simulink model "CCMDcMotor"  (R2022b)
settings: Solver=FixedStepDiscrete  StartTime=0.0  StopTime=10.0  FixedStep=0.01  RelTol=1e-3
model workspace (MAT data in the model file):
  NvMBlockDcMtrCalData = Simulink.Signal
  NvMBlockDcMtrCalData.DataType = 'UInt8'
  NvMBlockDcMtrCalData.Dimensions = [82 1]
  NvMBlockDcMtrCalData.DimensionsMode = 'auto'
  NvMBlockDcMtrCalData.Complexity = 'real'
  NvMBlockDcMtrCalData.SampleTime = -1
  NvMBlockDcMtrCalData.SamplingMode = 'auto'
  NvMBlockDcMtrCalData.InitialValue = 'NvMBlockDcMtrCalDataInit'
  NvMBlockDcMtrCalDataInit = Simulink.Parameter
  NvMBlockDcMtrCalDataInit.Value = [0; 0; 0; 0; 0; 0; 0; 0; 0; 0; 0; 0; 0; 0; 0; 0; 0; 0; 0; 0; 0; 0; 0; 0; 0; 0; 0; 0; 0; 0; 0; 0; 0; 0; 0; 0; 0; 0; 0; 0; 0; 0; 0; 0; 0; 0; 0; 0; 0; 0; 0; 0; 0; 0; 0; 0; 0; 0; 0; 0; 0; 0; 0; 0; 0; 0; 0; 0; 0; 0; 0; 0; 0; 0; 0; 0; 0; 0; 0; 0; 0; 0]
  NvMBlockDcMtrCalDataInit.DataType = 'UInt8'
  NvMBlockDcMtrCalDataInit.Complexity = 'real'
  NvMBlockDcMtrCalDataInit.Dimensions = [82 1]
undefined symbols (not defined in the file or in its folder): DTCState, NvMBlockId, P_Std_ReturnType, Tle91ChlType, Tle91CmdType
(part 1 of 3)
settings Model: Creator=blue.ge  LastModifiedBy=blue.ge
settings DataIOCC: SaveFormat=StructureWithTime
settings OptimizationCC: DefaultParameterBehavior=Inlined  EnhancedBackFolding=on  PassReuseOutputArgsAs=Individual arguments  ZeroExternalMemoryAtStartup=off  ZeroInternalMemoryAtStartup=off  OptimizeBlockOrder=speed  DifferentSizesBufferReuse=on  OptimizationCustomize=off  EfficientTunableParamExpr=on
settings DebuggingCC: ParameterTunabilityLossMsg=error
settings ModelReferenceCC: ModelReferenceNumInstancesAllowed=Single
settings SFSimCC: MATLABDynamicMemAlloc=off
settings RTWCC: Description=AUTOSAR  SystemTargetFile=autosar.tlc  GenCodeOnly=on  TemplateMakefile=ert_default_tmf
settings CodeAppCC: ForceParamTrailComments=on  IgnoreCustomStorageClasses=off  OperatorAnnotations=on  SimulinkDataObjDesc=on  SFDataObjDesc=on  InsertBlockDesc=on
settings STFCustomTargetCC: TargetLangStandard=C99 (ISO)  MultiwordLength=256  ParMdlRefBuildCompliant=on  CompOptLevelCompliant=on  IncludeMdlTerminateFcn=off  CombineOutputUpdateFcns=on  SuppressErrorStatus=on  ERTCustomFileBanners=on  SupportNonFinite=off  SupportComplex=off  SupportContinuousTime=off  SupportNonInlinedSFcns=off  RemoveResetFunc=on  MATLABClassNameForMDSCustomization=Simulink.SoftwareTarget.ERTCustomization  AutosarCompliant=on  UseToolchainInfoCompliant=on  LookupTableObjectStructAxisOrder=2,1,3,4,...  LUTObjectStructOrderEvenSpacing=Size,Table,Breakpoints  SystemTargetFile=autosar.tlc
settings ERTTargetCC: TargetLangStandard=C99 (ISO)  ConcurrentExecutionCompliant=off  IncludeMdlTerminateFcn=off  SuppressErrorStatus=on  SupportNonFinite=off  SupportComplex=off  ModelStepFunctionPrototypeControlCompliant=off  CPPClassGenCompliant=off  AutosarCompliant=on  LookupTableObjectStructAxisOrder=2,1,3,4,...  LUTObjectStructOrderEvenSpacing=Size,Table,Breakpoints  InlinedParameterPlacement=NonHierarchical  ERTCustomFileTemplate=example_file_process.tlc

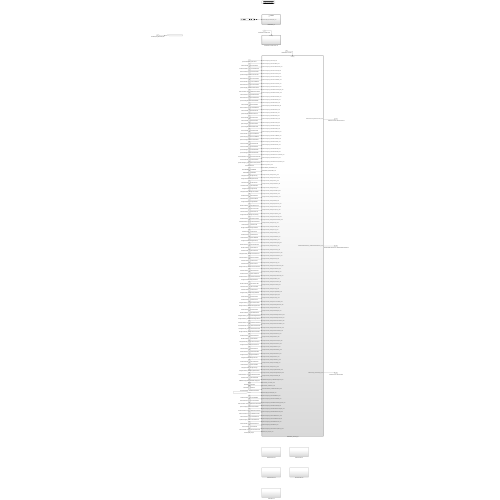
[thumbnail: Simulink's stored preview of the top-level diagram]
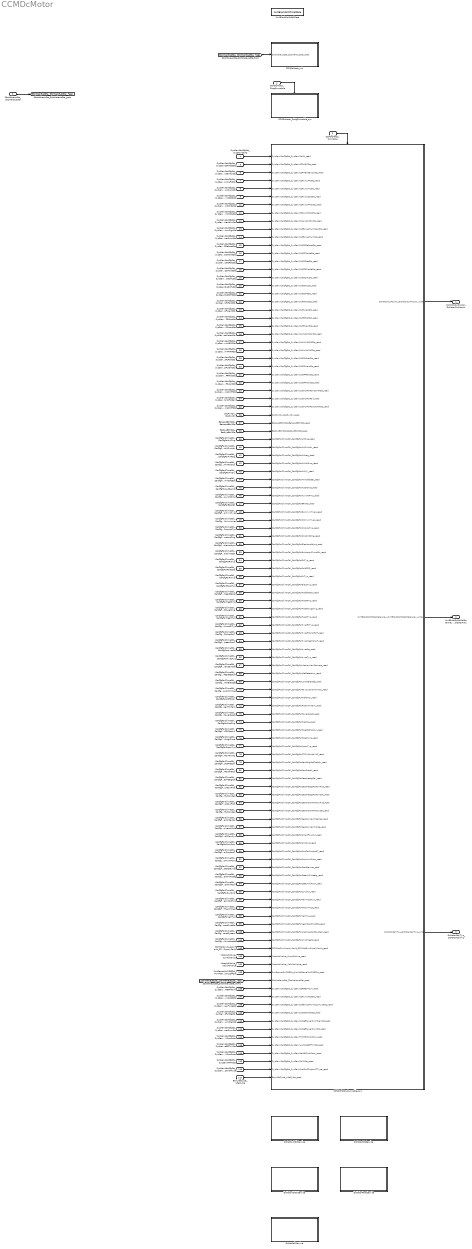
== system CCMDcMotor ==
  CCMDcMotor_DiagRunnable : Inport  SampleTime=0.05  OutputFunctionCall=on
  CCMDcMotor_Runnable : Inport  Port=2  SampleTime=0.01  OutputFunctionCall=on
  SbcWakeupSta_SbcWakeupSta : Inport  Port=3  OutDataTypeStr=Enum: SbcWakeupSta  PortDimensions=1
  SysServCarCfgSts_SysServCarID : Inport  Port=4  OutDataTypeStr=Enum: SysServCarID  PortDimensions=1
  SysServCarCfgSts_SysServCCPAQSSts : Inport  Port=5  OutDataTypeStr=UInt8  PortDimensions=1
  SysServCarCfgSts_SysServCCPHEXSensorSts : Inport  Port=6  OutDataTypeStr=UInt8  PortDimensions=1
  SysServCarCfgSts_SysServCCPLin2AFUSts : Inport  Port=7  OutDataTypeStr=Boolean  PortDimensions=1
  SysServCarCfgSts_SysServCCPLin2AIUSts : Inport  Port=8  OutDataTypeStr=UInt8  PortDimensions=1
  SysServCarCfgSts_SysServCCPLin2CDSSts : Inport  Port=9  OutDataTypeStr=Boolean  PortDimensions=1
  SysServCarCfgSts_SysServCCPLin2PMSISts : Inport  Port=10  OutDataTypeStr=Boolean  PortDimensions=1
  SysServCarCfgSts_SysServCCPLin3VGASts : Inport  Port=11  OutDataTypeStr=Boolean  PortDimensions=1
  SysServCarCfgSts_SysServDUAirVentSnrSts : Inport  Port=12  OutDataTypeStr=Boolean  PortDimensions=1
  SysServCarCfgSts_SysServDUBlowerPwmSignSts : Inport  Port=13  OutDataTypeStr=Boolean  PortDimensions=1
  SysServCarCfgSts_SysServDUBlowerPwmSts : Inport  Port=14  OutDataTypeStr=Boolean  PortDimensions=1
  SysServCarCfgSts_SysServDUDCDefrostSts : Inport  Port=15  OutDataTypeStr=UInt8  PortDimensions=1
  SysServCarCfgSts_SysServDUDCModeSts : Inport  Port=16  OutDataTypeStr=UInt8  PortDimensions=1
  SysServCarCfgSts_SysServDUDCRecSts : Inport  Port=17  OutDataTypeStr=UInt8  PortDimensions=1
  SysServCarCfgSts_SysServDUDCSModeSts : Inport  Port=18  OutDataTypeStr=UInt8  PortDimensions=1
  SysServCarCfgSts_SysServDUDCSTLSts : Inport  Port=19  OutDataTypeStr=UInt8  PortDimensions=1
  SysServCarCfgSts_SysServDUDCTLSts : Inport  Port=20  OutDataTypeStr=UInt8  PortDimensions=1
  SysServCarCfgSts_SysServDUDCTRSts : Inport  Port=21  OutDataTypeStr=UInt8  PortDimensions=1
  SysServCarCfgSts_SysServDUFLFootSts : Inport  Port=22  OutDataTypeStr=UInt8  PortDimensions=1
  SysServCarCfgSts_SysServDUFLVentSts : Inport  Port=23  OutDataTypeStr=UInt8  PortDimensions=1
  SysServCarCfgSts_SysServDUFRFootSts : Inport  Port=24  OutDataTypeStr=UInt8  PortDimensions=1
  SysServCarCfgSts_SysServDUFRVentSts : Inport  Port=25  OutDataTypeStr=UInt8  PortDimensions=1
  SysServCarCfgSts_SysServDUIncarMotorSts : Inport  Port=26  OutDataTypeStr=Boolean  PortDimensions=1
  SysServCarCfgSts_SysServDULin1HBMFSts : Inport  Port=27  OutDataTypeStr=Boolean  PortDimensions=1
  SysServCarCfgSts_SysServDULin4HFAFSts : Inport  Port=28  OutDataTypeStr=UInt32  PortDimensions=1
  SysServCarCfgSts_SysServDUSFLFootSts : Inport  Port=29  OutDataTypeStr=UInt8  PortDimensions=1
  SysServCarCfgSts_SysServDUSFLVentSts : Inport  Port=30  OutDataTypeStr=UInt8  PortDimensions=1
  SysServCarCfgSts_SysServDUSFRFootSts : Inport  Port=31  OutDataTypeStr=UInt8  PortDimensions=1
  SysServCarCfgSts_SysServDUSFRVentSts : Inport  Port=32  OutDataTypeStr=UInt8  PortDimensions=1
  SysServCarCfgSts_SysServDUSnsrForFCmptmtTSts : Inport  Port=33  OutDataTypeStr=UInt8  PortDimensions=1
  SysServCarCfgSts_SysServDUSnsrForRair : Inport  Port=34  OutDataTypeStr=UInt8  PortDimensions=1
  SysServCarCfgSts_SysServDUSnsrForRCmptmtTSts : Inport  Port=35  OutDataTypeStr=UInt8  PortDimensions=1
  SbcPwrOn_SbcPwrOn : Inport  Port=36  OutDataTypeStr=PwrOnff  PortDimensions=1
  BatLoadBtrMSts_BatLoadBtrMSts : Inport  Port=37  OutDataTypeStr=Enum: BatLoadBtrMStsEnum  PortDimensions=1
  BatSysBtrMSts_BatSysBtrMSts : Inport  Port=38  OutDataTypeStr=Enum: BatBtrMStsEnum  PortDimensions=1
  CarCfgForClimaCtrl_CarCfgForAirClng : Inport  Port=39  OutDataTypeStr=Enum: CarCfgForAirClng  PortDimensions=1
  CarCfgForClimaCtrl_CarCfgForAirClnrAdv : Inport  Port=40  OutDataTypeStr=UInt8  PortDimensions=1
  CarCfgForClimaCtrl_CarCfgForAirFrag : Inport  Port=41  OutDataTypeStr=Enum: CarCfgForAirFrag  PortDimensions=1
  CarCfgForClimaCtrl_CarCfgForAirMtrlSnsr : Inport  Port=42  OutDataTypeStr=Enum: CarCfgForAirMtrlSnsrGly  PortDimensions=1
  CarCfgForClimaCtrl_CarCfgForAirQly : Inport  Port=43  OutDataTypeStr=Enum: CarCfgForAirQlyGly  PortDimensions=1
  CarCfgForClimaCtrl_CarCfgForAmntOfSeat : Inport  Port=44  OutDataTypeStr=Enum: CarCfgForAmntOfSeatTyp3  PortDimensions=1
  CarCfgForClimaCtrl_CarCfgForAutDemst : Inport  Port=45  OutDataTypeStr=UInt8  PortDimensions=1
  CarCfgForClimaCtrl_CarCfgForAuxWtrPmp : Inport  Port=46  OutDataTypeStr=Enum: CarCfgForAuxWtrPmp  PortDimensions=1
  CarCfgForClimaCtrl_CarCfgForBRAND : Inport  Port=47  OutDataTypeStr=UInt8  PortDimensions=1
  CarCfgForClimaCtrl_CarCfgForBypVlvInHvac : Inport  Port=48  OutDataTypeStr=Enum: CarCfgForBypVlvInHvac2  PortDimensions=1
  CarCfgForClimaCtrl_CarCfgForClsVlvInHvac : Inport  Port=49  OutDataTypeStr=Enum: CarCfgForClsVlvInHvac  PortDimensions=1
  CarCfgForClimaCtrl_CarCfgForCmprAcTyp : Inport  Port=50  OutDataTypeStr=UInt8  PortDimensions=1
  CarCfgForClimaCtrl_CarCfgForCmptmtClng : Inport  Port=51  OutDataTypeStr=Enum: CarCfgForCmptmtClng  PortDimensions=1
  CarCfgForClimaCtrl_CarCfgForElecVentAdjmt : Inport  Port=52  OutDataTypeStr=Enum: CarCfgForElecVentAdjmt  PortDimensions=1
  CarCfgForClimaCtrl_CarCfgForExtVentnClimaCtrl : Inport  Port=53  OutDataTypeStr=Enum: CarCfgForExtVentnClimaCtrl  PortDimensions=1
  CarCfgForClimaCtrl_CarCfgForFlcTyp : Inport  Port=54  OutDataTypeStr=Enum: CarCfgForFlcTyp  PortDimensions=1
  CarCfgForClimaCtrl_CarCfgForFoldCSD : Inport  Port=55  OutDataTypeStr=Enum: CarCfgForFoldCSD  PortDimensions=1
  CarCfgForClimaCtrl_CarCfgForFuTyp : Inport  Port=56  OutDataTypeStr=Enum: CarCfgForFuTyp  PortDimensions=1
  CarCfgForClimaCtrl_CarCfgForGlassTyp : Inport  Port=57  OutDataTypeStr=Enum: CarCfgForGlassTyp  PortDimensions=1
  CarCfgForClimaCtrl_CarCfgForHeadRadar : Inport  Port=58  OutDataTypeStr=Enum: HeadRadar  PortDimensions=1
  CarCfgForClimaCtrl_CarCfgForHeatPmp : Inport  Port=59  OutDataTypeStr=Enum: CarCfgForHeatPmpTyp  PortDimensions=1
  CarCfgForClimaCtrl_CarCfgForHeatrDurgDrvg : Inport  Port=60  OutDataTypeStr=Enum: CarCfgForHeatrDurgDrvg  PortDimensions=1
  CarCfgForClimaCtrl_CarCfgForHeatrTyp : Inport  Port=61  OutDataTypeStr=UInt8  PortDimensions=1
  CarCfgForClimaCtrl_CarCfgForHvacFilTyp : Inport  Port=62  OutDataTypeStr=Enum: CarCfgForHvacFilTypGly  PortDimensions=1
  CarCfgForClimaCtrl_CarCfgForHvacFlrSnsrForT : Inport  Port=63  OutDataTypeStr=Enum: CarCfgForHvacFlrSnsrForT  PortDimensions=1
  CarCfgForClimaCtrl_CarCfgForHvacHeatrSnsrT : Inport  Port=64  OutDataTypeStr=Enum: CarCfgForHvacHeatrSnsrT  PortDimensions=1
  CarCfgForClimaCtrl_CarCfgForHvacRe : Inport  Port=65  OutDataTypeStr=UInt8  PortDimensions=1
  CarCfgForClimaCtrl_CarCfgForHvacTyp : Inport  Port=66  OutDataTypeStr=UInt8  PortDimensions=1
  CarCfgForClimaCtrl_CarCfgForInteriorViewCamera : Inport  Port=67  OutDataTypeStr=Enum: CarCfgForInteriorViewCamera  PortDimensions=1
  CarCfgForClimaCtrl_CarCfgForLifeDetection : Inport  Port=68  OutDataTypeStr=Enum: LifeDetection  PortDimensions=1
  CarCfgForClimaCtrl_CarCfgForLoVoltElecCfg : Inport  Port=69  OutDataTypeStr=Enum: CarCfgForLoVoltElecCfg  PortDimensions=1
  CarCfgForClimaCtrl_CarCfgForNavSysandIntnmap : Inport  Port=70  OutDataTypeStr=Enum: CarCfgForNavSysandIntnmap  PortDimensions=1
  CarCfgForClimaCtrl_CarCfgForNrOfDoor : Inport  Port=71  OutDataTypeStr=Enum: CarCfgForNrOfDoor2  PortDimensions=1
  CarCfgForClimaCtrl_CarCfgForPipeInFuTank : Inport  Port=72  OutDataTypeStr=Enum: CarCfgForPipeInFuTank  PortDimensions=1
  CarCfgForClimaCtrl_CarCfgForPowerSeats : Inport  Port=73  OutDataTypeStr=Enum: CarCfgForPowerSeats  PortDimensions=1
  CarCfgForClimaCtrl_CarCfgForPreClng : Inport  Port=74  OutDataTypeStr=Enum: CarCfgForPreClng  PortDimensions=1
  CarCfgForClimaCtrl_CarCfgForPrkgCDSNotAvl : Inport  Port=75  OutDataTypeStr=Enum: CarCfgForPrkgCDSNotAvl  PortDimensions=1
  CarCfgForClimaCtrl_CarCfgForPrkgClima : Inport  Port=76  OutDataTypeStr=Enum: CarCfgForPrkgClima  PortDimensions=1
  CarCfgForClimaCtrl_CarCfgForPrpsnTyp : Inport  Port=77  OutDataTypeStr=Enum: CarCfgForPrpsnTypGly  PortDimensions=1
  CarCfgForClimaCtrl_CarCfgForPTCInFrontHVAC : Inport  Port=78  OutDataTypeStr=UInt8  PortDimensions=1
  CarCfgForClimaCtrl_CarCfgForRemEngStrtPreCdn : Inport  Port=79  OutDataTypeStr=Enum: CarCfgForRemEngStrtPreCdn  PortDimensions=1
  CarCfgForClimaCtrl_CarCfgForRoofHatch : Inport  Port=80  OutDataTypeStr=Enum: CarCfgForRoofHatch  PortDimensions=1
  CarCfgForClimaCtrl_CarCfgForSeatHeatgCal : Inport  Port=81  OutDataTypeStr=Enum: CarCfgForSeatHeatgCal1  PortDimensions=1
  CarCfgForClimaCtrl_CarCfgForSeatHeatgForRowFirst : Inport  Port=82  OutDataTypeStr=Enum: CarCfgForSeatHeatgForRowFirst  PortDimensions=1
  CarCfgForClimaCtrl_CarCfgForSeatHeatgForRowSec : Inport  Port=83  OutDataTypeStr=Enum: CarCfgForSeatHeatgForRowSec  PortDimensions=1
  CarCfgForClimaCtrl_CarCfgForSeatVentnForRowFirst : Inport  Port=84  OutDataTypeStr=Enum: CarCfgForSeatVentnForRowFirst1  PortDimensions=1
  CarCfgForClimaCtrl_CarCfgForSeatVentnForRowSec : Inport  Port=85  OutDataTypeStr=Enum: CarCfgForSeatVentnForRowSecGly  PortDimensions=1
  CarCfgForClimaCtrl_CarCfgForSecRowVentnCenter : Inport  Port=86  OutDataTypeStr=Enum: CarCfgForSecRowVentnCenter  PortDimensions=1
  CarCfgForClimaCtrl_CarCfgForSecRowVentnSide : Inport  Port=87  OutDataTypeStr=Enum: CarCfgForSecRowVentnSide  PortDimensions=1
  CarCfgForClimaCtrl_CarCfgForSmallFlowCyc : Inport  Port=88  OutDataTypeStr=Enum: CarCfgForSmallFlowCyc  PortDimensions=1
  CarCfgForClimaCtrl_CarCfgForSmkSnsr : Inport  Port=89  OutDataTypeStr=Enum: CarCfgForSmkSnsr  PortDimensions=1
  CarCfgForClimaCtrl_CarCfgForSnsrForCmptmtT : Inport  Port=90  OutDataTypeStr=Enum: CarCfgForSnsrForCmptmtT  PortDimensions=1
  CarCfgForClimaCtrl_CarCfgForSnsrHumPrsnt : Inport  Port=91  OutDataTypeStr=Enum: CarCfgForSnsrHumPrsnt  PortDimensions=1
  CarCfgForClimaCtrl_CarCfgForStartDevice : Inport  Port=92  OutDataTypeStr=Enum: StartDevice  PortDimensions=1
  CarCfgForClimaCtrl_CarCfgForSteerWhlHeatg : Inport  Port=93  OutDataTypeStr=UInt8  PortDimensions=1
  CarCfgForClimaCtrl_CarCfgForSteerWhlPosn : Inport  Port=94  OutDataTypeStr=Enum: CarCfgForSteerWhlPosn  PortDimensions=1
  CarCfgForClimaCtrl_CarCfgForSunSnsr : Inport  Port=95  OutDataTypeStr=Enum: CarCfgForSunSnsr  PortDimensions=1
  CarCfgForClimaCtrl_CarCfgForTelmNodTyp : Inport  Port=96  OutDataTypeStr=Enum: CarCfgForTelmNodTyp  PortDimensions=1
  CarCfgForClimaCtrl_CarCfgForThermMngt : Inport  Port=97  OutDataTypeStr=Enum: CarCfgForThermMngt  PortDimensions=1
  CarCfgForClimaCtrl_CarCfgForVehTyp : Inport  Port=98  OutDataTypeStr=Enum: CarCfgForVehTyp3  PortDimensions=1
  CarCfgForClimaCtrl_CarCfgForVgaMotorExistSts : Inport  Port=99  OutDataTypeStr=CarCfgForVgaMotorExistSts  PortDimensions=1
  CarCfgForClimaCtrl_CarCfgForWadingAssistSystem : Inport  Port=100  OutDataTypeStr=UInt8  PortDimensions=1
  CarCfgForClimaCtrl_CarCfgForWindHeatd : Inport  Port=101  OutDataTypeStr=Enum: CarCfgForWindHeatd  PortDimensions=1
  CCMMotDrvrSupplyFault_CCMMotDrvrSupplyFault : Inport  Port=102  OutDataTypeStr=Enum: ChargePump  PortDimensions=1
  MotorAdValue_CurrAdValue : Inport  Port=103  OutDataTypeStr=Bus: MotorAdValueType  PortDimensions=1  BusOutputAsStruct=on
  MotorAdValue_ValidAdValue : Inport  Port=104  OutDataTypeStr=Bus: MotorAdValueType  PortDimensions=1  BusOutputAsStruct=on
  PwrGernalOn2OffCnt_PwrMdGernalOn2OffCnt : Inport  Port=105  OutDataTypeStr=On2OffCnt  PortDimensions=1
  SysServCarCfgSts_SysServCCPBGMNum : Inport  Port=106  OutDataTypeStr=UInt8  PortDimensions=1
  SysServCarCfgSts_SysServCCPLin2AQSSts : Inport  Port=107  OutDataTypeStr=Boolean  PortDimensions=1
  SysServCarCfgSts_SysServDUBlowerPwmSignTypeSts : Inport  Port=108  OutDataTypeStr=UInt8  PortDimensions=1
  SysServCarCfgSts_SysServDUDCFLMdeSts : Inport  Port=109  OutDataTypeStr=UInt8  PortDimensions=1
  SysServCarCfgSts_SysServDUReBlowerPwmSignSts : Inport  Port=110  OutDataTypeStr=UInt8  PortDimensions=1
  SysServCarCfgSts_SysServDUReBlowerPwmSts : Inport  Port=111  OutDataTypeStr=UInt8  PortDimensions=1
  SysServCarCfgSts_SysServFrntHEXSnsrPosn : Inport  Port=112  OutDataTypeStr=UInt8  PortDimensions=1
  SysServCarCfgSts_SysServLowSideRETXVSts : Inport  Port=113  OutDataTypeStr=UInt8  PortDimensions=1
  SysServCarCfgSts_SysServReHEXSnsrPosn : Inport  Port=114  OutDataTypeStr=UInt8  PortDimensions=1
  SysServCarCfgSts_SysServRHFASts : Inport  Port=115  OutDataTypeStr=UInt8  PortDimensions=1
  SysServCarCfgSts_SysServSnsrForFCmptmtTType : Inport  Port=116  OutDataTypeStr=UInt8  PortDimensions=1
  BswLifeCycle_LifeCycle : Inport  Port=117  OutDataTypeStr=Enum: CCMLifeCycleModeGroup  PortDimensions=1
  CCMDcMotor_DiagRunnable_sys : Subsystem
  CCMDcMotor_Init : Subsystem
  CCMDcMotor_Runnable_sys : Subsystem
  DcMotNvMClrService : Subsystem
  DcMotorDtcService : Subsystem
  DcMotorIOCtrlService : Subsystem
  DcMotorParaService : Subsystem
  DcMtrCaliServive : Subsystem
  NvMBlockDcMtrCalData : DataStoreMemory  DataStoreName=NvMBlockDcMtrCalData  InitialValue=NvMBlockDcMtrCalDataInit  ShowAdditionalParam=off  Dimensions=82  StateMustResolveToSignalObject=on
  DcMotorCurPosVol_DcMotorCurPosVol : Outport  OutDataTypeStr=UInt16  PortDimensions=7
  NvMBlockDcMtrCalDataArray_NvMBlockDcMtrCalDataArray : Outport  Port=2  OutDataTypeStr=UInt8  PortDimensions=82
  DcMotorCaliTyp_DcMotorCaliTyp : Outport  Port=3  OutDataTypeStr=UInt8  PortDimensions=1
  wires:
  SysServCarCfgSts_SysServCarID/1 -> CCMDcMotor_Runnable_sys/1(SysServCarCfgSts_SysServCarID_read)
  SysServCarCfgSts_SysServCCPAQSSts/1 -> CCMDcMotor_Runnable_sys/2(SysServCarCfgSts_SysServCCPAQSSts_read)
  SysServCarCfgSts_SysServCCPHEXSensorSts/1 -> CCMDcMotor_Runnable_sys/3(SysServCarCfgSts_SysServCCPHEXSensorSts_read)
  SysServCarCfgSts_SysServCCPLin2AFUSts/1 -> CCMDcMotor_Runnable_sys/4(SysServCarCfgSts_SysServCCPLin2AFUSts_read)
  SysServCarCfgSts_SysServCCPLin2AIUSts/1 -> CCMDcMotor_Runnable_sys/5(SysServCarCfgSts_SysServCCPLin2AIUSts_read)
  SysServCarCfgSts_SysServCCPLin2CDSSts/1 -> CCMDcMotor_Runnable_sys/6(SysServCarCfgSts_SysServCCPLin2CDSSts_read)
  SysServCarCfgSts_SysServCCPLin2PMSISts/1 -> CCMDcMotor_Runnable_sys/7(SysServCarCfgSts_SysServCCPLin2PMSISts_read)
  SysServCarCfgSts_SysServCCPLin3VGASts/1 -> CCMDcMotor_Runnable_sys/8(SysServCarCfgSts_SysServCCPLin3VGASts_read)
  SysServCarCfgSts_SysServDUAirVentSnrSts/1 -> CCMDcMotor_Runnable_sys/9(SysServCarCfgSts_SysServDUAirVentSnrSts_read)
  SysServCarCfgSts_SysServDUBlowerPwmSignSts/1 -> CCMDcMotor_Runnable_sys/10(SysServCarCfgSts_SysServDUBlowerPwmSignSts_read)
  SysServCarCfgSts_SysServDUBlowerPwmSts/1 -> CCMDcMotor_Runnable_sys/11(SysServCarCfgSts_SysServDUBlowerPwmSts_read)
  SysServCarCfgSts_SysServDUDCDefrostSts/1 -> CCMDcMotor_Runnable_sys/12(SysServCarCfgSts_SysServDUDCDefrostSts_read)
  SysServCarCfgSts_SysServDUDCModeSts/1 -> CCMDcMotor_Runnable_sys/13(SysServCarCfgSts_SysServDUDCModeSts_read)
  SysServCarCfgSts_SysServDUDCRecSts/1 -> CCMDcMotor_Runnable_sys/14(SysServCarCfgSts_SysServDUDCRecSts_read)
  SysServCarCfgSts_SysServDUDCSModeSts/1 -> CCMDcMotor_Runnable_sys/15(SysServCarCfgSts_SysServDUDCSModeSts_read)
  SysServCarCfgSts_SysServDUDCSTLSts/1 -> CCMDcMotor_Runnable_sys/16(SysServCarCfgSts_SysServDUDCSTLSts_read)
  SysServCarCfgSts_SysServDUDCTLSts/1 -> CCMDcMotor_Runnable_sys/17(SysServCarCfgSts_SysServDUDCTLSts_read)
  SysServCarCfgSts_SysServDUDCTRSts/1 -> CCMDcMotor_Runnable_sys/18(SysServCarCfgSts_SysServDUDCTRSts_read)
  SysServCarCfgSts_SysServDUFLFootSts/1 -> CCMDcMotor_Runnable_sys/19(SysServCarCfgSts_SysServDUFLFootSts_read)
  SysServCarCfgSts_SysServDUFLVentSts/1 -> CCMDcMotor_Runnable_sys/20(SysServCarCfgSts_SysServDUFLVentSts_read)
  SysServCarCfgSts_SysServDUFRFootSts/1 -> CCMDcMotor_Runnable_sys/21(SysServCarCfgSts_SysServDUFRFootSts_read)
  SysServCarCfgSts_SysServDUFRVentSts/1 -> CCMDcMotor_Runnable_sys/22(SysServCarCfgSts_SysServDUFRVentSts_read)
  SysServCarCfgSts_SysServDUIncarMotorSts/1 -> CCMDcMotor_Runnable_sys/23(SysServCarCfgSts_SysServDUIncarMotorSts_read)
  SysServCarCfgSts_SysServDULin1HBMFSts/1 -> CCMDcMotor_Runnable_sys/24(SysServCarCfgSts_SysServDULin1HBMFSts_read)
  SysServCarCfgSts_SysServDULin4HFAFSts/1 -> CCMDcMotor_Runnable_sys/25(SysServCarCfgSts_SysServDULin4HFAFSts_read)
  SysServCarCfgSts_SysServDUSFLFootSts/1 -> CCMDcMotor_Runnable_sys/26(SysServCarCfgSts_SysServDUSFLFootSts_read)
  SysServCarCfgSts_SysServDUSFLVentSts/1 -> CCMDcMotor_Runnable_sys/27(SysServCarCfgSts_SysServDUSFLVentSts_read)
  SysServCarCfgSts_SysServDUSFRFootSts/1 -> CCMDcMotor_Runnable_sys/28(SysServCarCfgSts_SysServDUSFRFootSts_read)
  SysServCarCfgSts_SysServDUSFRVentSts/1 -> CCMDcMotor_Runnable_sys/29(SysServCarCfgSts_SysServDUSFRVentSts_read)
  SysServCarCfgSts_SysServDUSnsrForFCmptmtTSts/1 -> CCMDcMotor_Runnable_sys/30(SysServCarCfgSts_SysServDUSnsrForFCmptmtTSts_read)
  SysServCarCfgSts_SysServDUSnsrForRair/1 -> CCMDcMotor_Runnable_sys/31(SysServCarCfgSts_SysServDUSnsrForRair_read)
  SysServCarCfgSts_SysServDUSnsrForRCmptmtTSts/1 -> CCMDcMotor_Runnable_sys/32(SysServCarCfgSts_SysServDUSnsrForRCmptmtTSts_read)
  SbcPwrOn_SbcPwrOn/1 -> CCMDcMotor_Runnable_sys/33(SbcPwrOn_SbcPwrOn_read)
  BatLoadBtrMSts_BatLoadBtrMSts/1 -> CCMDcMotor_Runnable_sys/34(BatLoadBtrMSts_BatLoadBtrMSts_read)
  BatSysBtrMSts_BatSysBtrMSts/1 -> CCMDcMotor_Runnable_sys/35(BatSysBtrMSts_BatSysBtrMSts_read)
  CarCfgForClimaCtrl_CarCfgForAirClng/1 -> CCMDcMotor_Runnable_sys/36(CarCfgForClimaCtrl_CarCfgForAirClng_read)
  CarCfgForClimaCtrl_CarCfgForAirClnrAdv/1 -> CCMDcMotor_Runnable_sys/37(CarCfgForClimaCtrl_CarCfgForAirClnrAdv_read)
  CarCfgForClimaCtrl_CarCfgForAirFrag/1 -> CCMDcMotor_Runnable_sys/38(CarCfgForClimaCtrl_CarCfgForAirFrag_read)
  CarCfgForClimaCtrl_CarCfgForAirMtrlSnsr/1 -> CCMDcMotor_Runnable_sys/39(CarCfgForClimaCtrl_CarCfgForAirMtrlSnsr_read)
  CarCfgForClimaCtrl_CarCfgForAirQly/1 -> CCMDcMotor_Runnable_sys/40(CarCfgForClimaCtrl_CarCfgForAirQly_read)
  CarCfgForClimaCtrl_CarCfgForAmntOfSeat/1 -> CCMDcMotor_Runnable_sys/41(CarCfgForClimaCtrl_CarCfgForAmntOfSeat_read)
  CarCfgForClimaCtrl_CarCfgForAutDemst/1 -> CCMDcMotor_Runnable_sys/42(CarCfgForClimaCtrl_CarCfgForAutDemst_read)
  CarCfgForClimaCtrl_CarCfgForAuxWtrPmp/1 -> CCMDcMotor_Runnable_sys/43(CarCfgForClimaCtrl_CarCfgForAuxWtrPmp_read)
  CarCfgForClimaCtrl_CarCfgForBRAND/1 -> CCMDcMotor_Runnable_sys/44(CarCfgForClimaCtrl_CarCfgForBRAND_read)
  CarCfgForClimaCtrl_CarCfgForBypVlvInHvac/1 -> CCMDcMotor_Runnable_sys/45(CarCfgForClimaCtrl_CarCfgForBypVlvInHvac_read)
  CarCfgForClimaCtrl_CarCfgForClsVlvInHvac/1 -> CCMDcMotor_Runnable_sys/46(CarCfgForClimaCtrl_CarCfgForClsVlvInHvac_read)
  CarCfgForClimaCtrl_CarCfgForCmprAcTyp/1 -> CCMDcMotor_Runnable_sys/47(CarCfgForClimaCtrl_CarCfgForCmprAcTyp_read)
  CarCfgForClimaCtrl_CarCfgForCmptmtClng/1 -> CCMDcMotor_Runnable_sys/48(CarCfgForClimaCtrl_CarCfgForCmptmtClng_read)
  CarCfgForClimaCtrl_CarCfgForElecVentAdjmt/1 -> CCMDcMotor_Runnable_sys/49(CarCfgForClimaCtrl_CarCfgForElecVentAdjmt_read)
  CarCfgForClimaCtrl_CarCfgForExtVentnClimaCtrl/1 -> CCMDcMotor_Runnable_sys/50(CarCfgForClimaCtrl_CarCfgForExtVentnClimaCtrl_read)
  CarCfgForClimaCtrl_CarCfgForFlcTyp/1 -> CCMDcMotor_Runnable_sys/51(CarCfgForClimaCtrl_CarCfgForFlcTyp_read)
  CarCfgForClimaCtrl_CarCfgForFoldCSD/1 -> CCMDcMotor_Runnable_sys/52(CarCfgForClimaCtrl_CarCfgForFoldCSD_read)
  CarCfgForClimaCtrl_CarCfgForFuTyp/1 -> CCMDcMotor_Runnable_sys/53(CarCfgForClimaCtrl_CarCfgForFuTyp_read)
  CarCfgForClimaCtrl_CarCfgForGlassTyp/1 -> CCMDcMotor_Runnable_sys/54(CarCfgForClimaCtrl_CarCfgForGlassTyp_read)
  CarCfgForClimaCtrl_CarCfgForHeadRadar/1 -> CCMDcMotor_Runnable_sys/55(CarCfgForClimaCtrl_CarCfgForHeadRadar_read)
  CarCfgForClimaCtrl_CarCfgForHeatPmp/1 -> CCMDcMotor_Runnable_sys/56(CarCfgForClimaCtrl_CarCfgForHeatPmp_read)
  CarCfgForClimaCtrl_CarCfgForHeatrDurgDrvg/1 -> CCMDcMotor_Runnable_sys/57(CarCfgForClimaCtrl_CarCfgForHeatrDurgDrvg_read)
  CarCfgForClimaCtrl_CarCfgForHeatrTyp/1 -> CCMDcMotor_Runnable_sys/58(CarCfgForClimaCtrl_CarCfgForHeatrTyp_read)
  CarCfgForClimaCtrl_CarCfgForHvacFilTyp/1 -> CCMDcMotor_Runnable_sys/59(CarCfgForClimaCtrl_CarCfgForHvacFilTyp_read)
  CarCfgForClimaCtrl_CarCfgForHvacFlrSnsrForT/1 -> CCMDcMotor_Runnable_sys/60(CarCfgForClimaCtrl_CarCfgForHvacFlrSnsrForT_read)
  CarCfgForClimaCtrl_CarCfgForHvacHeatrSnsrT/1 -> CCMDcMotor_Runnable_sys/61(CarCfgForClimaCtrl_CarCfgForHvacHeatrSnsrT_read)
  CarCfgForClimaCtrl_CarCfgForHvacRe/1 -> CCMDcMotor_Runnable_sys/62(CarCfgForClimaCtrl_CarCfgForHvacRe_read)
  CarCfgForClimaCtrl_CarCfgForHvacTyp/1 -> CCMDcMotor_Runnable_sys/63(CarCfgForClimaCtrl_CarCfgForHvacTyp_read)
  CarCfgForClimaCtrl_CarCfgForInteriorViewCamera/1 -> CCMDcMotor_Runnable_sys/64(CarCfgForClimaCtrl_CarCfgForInteriorViewCamera_read)
  CarCfgForClimaCtrl_CarCfgForLifeDetection/1 -> CCMDcMotor_Runnable_sys/65(CarCfgForClimaCtrl_CarCfgForLifeDetection_read)
  CarCfgForClimaCtrl_CarCfgForLoVoltElecCfg/1 -> CCMDcMotor_Runnable_sys/66(CarCfgForClimaCtrl_CarCfgForLoVoltElecCfg_read)
  CarCfgForClimaCtrl_CarCfgForNavSysandIntnmap/1 -> CCMDcMotor_Runnable_sys/67(CarCfgForClimaCtrl_CarCfgForNavSysandIntnmap_read)
  CarCfgForClimaCtrl_CarCfgForNrOfDoor/1 -> CCMDcMotor_Runnable_sys/68(CarCfgForClimaCtrl_CarCfgForNrOfDoor_read)
  CarCfgForClimaCtrl_CarCfgForPipeInFuTank/1 -> CCMDcMotor_Runnable_sys/69(CarCfgForClimaCtrl_CarCfgForPipeInFuTank_read)
  CarCfgForClimaCtrl_CarCfgForPowerSeats/1 -> CCMDcMotor_Runnable_sys/70(CarCfgForClimaCtrl_CarCfgForPowerSeats_read)
  CarCfgForClimaCtrl_CarCfgForPreClng/1 -> CCMDcMotor_Runnable_sys/71(CarCfgForClimaCtrl_CarCfgForPreClng_read)
  CarCfgForClimaCtrl_CarCfgForPrkgCDSNotAvl/1 -> CCMDcMotor_Runnable_sys/72(CarCfgForClimaCtrl_CarCfgForPrkgCDSNotAvl_read)
  CarCfgForClimaCtrl_CarCfgForPrkgClima/1 -> CCMDcMotor_Runnable_sys/73(CarCfgForClimaCtrl_CarCfgForPrkgClima_read)
  CarCfgForClimaCtrl_CarCfgForPrpsnTyp/1 -> CCMDcMotor_Runnable_sys/74(CarCfgForClimaCtrl_CarCfgForPrpsnTyp_read)
  CarCfgForClimaCtrl_CarCfgForPTCInFrontHVAC/1 -> CCMDcMotor_Runnable_sys/75(CarCfgForClimaCtrl_CarCfgForPTCInFrontHVAC_read)
  CarCfgForClimaCtrl_CarCfgForRemEngStrtPreCdn/1 -> CCMDcMotor_Runnable_sys/76(CarCfgForClimaCtrl_CarCfgForRemEngStrtPreCdn_read)
  CarCfgForClimaCtrl_CarCfgForRoofHatch/1 -> CCMDcMotor_Runnable_sys/77(CarCfgForClimaCtrl_CarCfgForRoofHatch_read)
  CarCfgForClimaCtrl_CarCfgForSeatHeatgCal/1 -> CCMDcMotor_Runnable_sys/78(CarCfgForClimaCtrl_CarCfgForSeatHeatgCal_read)
  CarCfgForClimaCtrl_CarCfgForSeatHeatgForRowFirst/1 -> CCMDcMotor_Runnable_sys/79(CarCfgForClimaCtrl_CarCfgForSeatHeatgForRowFirst_read)
  CarCfgForClimaCtrl_CarCfgForSeatHeatgForRowSec/1 -> CCMDcMotor_Runnable_sys/80(CarCfgForClimaCtrl_CarCfgForSeatHeatgForRowSec_read)
  CarCfgForClimaCtrl_CarCfgForSeatVentnForRowFirst/1 -> CCMDcMotor_Runnable_sys/81(CarCfgForClimaCtrl_CarCfgForSeatVentnForRowFirst_read)
  CarCfgForClimaCtrl_CarCfgForSeatVentnForRowSec/1 -> CCMDcMotor_Runnable_sys/82(CarCfgForClimaCtrl_CarCfgForSeatVentnForRowSec_read)
  CarCfgForClimaCtrl_CarCfgForSecRowVentnCenter/1 -> CCMDcMotor_Runnable_sys/83(CarCfgForClimaCtrl_CarCfgForSecRowVentnCenter_read)
  CarCfgForClimaCtrl_CarCfgForSecRowVentnSide/1 -> CCMDcMotor_Runnable_sys/84(CarCfgForClimaCtrl_CarCfgForSecRowVentnSide_read)
  CarCfgForClimaCtrl_CarCfgForSmallFlowCyc/1 -> CCMDcMotor_Runnable_sys/85(CarCfgForClimaCtrl_CarCfgForSmallFlowCyc_read)
  CarCfgForClimaCtrl_CarCfgForSmkSnsr/1 -> CCMDcMotor_Runnable_sys/86(CarCfgForClimaCtrl_CarCfgForSmkSnsr_read)
  CarCfgForClimaCtrl_CarCfgForSnsrForCmptmtT/1 -> CCMDcMotor_Runnable_sys/87(CarCfgForClimaCtrl_CarCfgForSnsrForCmptmtT_read)
  CarCfgForClimaCtrl_CarCfgForSnsrHumPrsnt/1 -> CCMDcMotor_Runnable_sys/88(CarCfgForClimaCtrl_CarCfgForSnsrHumPrsnt_read)
  CarCfgForClimaCtrl_CarCfgForStartDevice/1 -> CCMDcMotor_Runnable_sys/89(CarCfgForClimaCtrl_CarCfgForStartDevice_read)
  CarCfgForClimaCtrl_CarCfgForSteerWhlHeatg/1 -> CCMDcMotor_Runnable_sys/90(CarCfgForClimaCtrl_CarCfgForSteerWhlHeatg_read)
  CarCfgForClimaCtrl_CarCfgForSteerWhlPosn/1 -> CCMDcMotor_Runnable_sys/91(CarCfgForClimaCtrl_CarCfgForSteerWhlPosn_read)
  CarCfgForClimaCtrl_CarCfgForSunSnsr/1 -> CCMDcMotor_Runnable_sys/92(CarCfgForClimaCtrl_CarCfgForSunSnsr_read)
  CarCfgForClimaCtrl_CarCfgForTelmNodTyp/1 -> CCMDcMotor_Runnable_sys/93(CarCfgForClimaCtrl_CarCfgForTelmNodTyp_read)
  CarCfgForClimaCtrl_CarCfgForThermMngt/1 -> CCMDcMotor_Runnable_sys/94(CarCfgForClimaCtrl_CarCfgForThermMngt_read)
  CarCfgForClimaCtrl_CarCfgForVehTyp/1 -> CCMDcMotor_Runnable_sys/95(CarCfgForClimaCtrl_CarCfgForVehTyp_read)
  CarCfgForClimaCtrl_CarCfgForVgaMotorExistSts/1 -> CCMDcMotor_Runnable_sys/96(CarCfgForClimaCtrl_CarCfgForVgaMotorExistSts_read)
  CarCfgForClimaCtrl_CarCfgForWadingAssistSystem/1 -> CCMDcMotor_Runnable_sys/97(CarCfgForClimaCtrl_CarCfgForWadingAssistSystem_read)
  CarCfgForClimaCtrl_CarCfgForWindHeatd/1 -> CCMDcMotor_Runnable_sys/98(CarCfgForClimaCtrl_CarCfgForWindHeatd_read)
  CCMMotDrvrSupplyFault_CCMMotDrvrSupplyFault/1 -> CCMDcMotor_Runnable_sys/99(CCMMotDrvrSupplyFault_CCMMotDrvrSupplyFault_read)
  MotorAdValue_CurrAdValue/1 -> CCMDcMotor_Runnable_sys/100(MotorAdValue_CurrAdValue_read)
  MotorAdValue_ValidAdValue/1 -> CCMDcMotor_Runnable_sys/101(MotorAdValue_ValidAdValue_read)
  PwrGernalOn2OffCnt_PwrMdGernalOn2OffCnt/1 -> CCMDcMotor_Runnable_sys/102(PwrGernalOn2OffCnt_PwrMdGernalOn2OffCnt_read)
  SysServCarCfgSts_SysServCCPBGMNum/1 -> CCMDcMotor_Runnable_sys/104(SysServCarCfgSts_SysServCCPBGMNum_read)
  SysServCarCfgSts_SysServCCPLin2AQSSts/1 -> CCMDcMotor_Runnable_sys/105(SysServCarCfgSts_SysServCCPLin2AQSSts_read)
  SysServCarCfgSts_SysServDUBlowerPwmSignTypeSts/1 -> CCMDcMotor_Runnable_sys/106(SysServCarCfgSts_SysServDUBlowerPwmSignTypeSts_read)
  SysServCarCfgSts_SysServDUDCFLMdeSts/1 -> CCMDcMotor_Runnable_sys/107(SysServCarCfgSts_SysServDUDCFLMdeSts_read)
  SysServCarCfgSts_SysServDUReBlowerPwmSignSts/1 -> CCMDcMotor_Runnable_sys/108(SysServCarCfgSts_SysServDUReBlowerPwmSignSts_read)
  SysServCarCfgSts_SysServDUReBlowerPwmSts/1 -> CCMDcMotor_Runnable_sys/109(SysServCarCfgSts_SysServDUReBlowerPwmSts_read)
  SysServCarCfgSts_SysServFrntHEXSnsrPosn/1 -> CCMDcMotor_Runnable_sys/110(SysServCarCfgSts_SysServFrntHEXSnsrPosn_read)
  SysServCarCfgSts_SysServLowSideRETXVSts/1 -> CCMDcMotor_Runnable_sys/111(SysServCarCfgSts_SysServLowSideRETXVSts_read)
  SysServCarCfgSts_SysServReHEXSnsrPosn/1 -> CCMDcMotor_Runnable_sys/112(SysServCarCfgSts_SysServReHEXSnsrPosn_read)
  SysServCarCfgSts_SysServRHFASts/1 -> CCMDcMotor_Runnable_sys/113(SysServCarCfgSts_SysServRHFASts_read)
  SysServCarCfgSts_SysServSnsrForFCmptmtTType/1 -> CCMDcMotor_Runnable_sys/114(SysServCarCfgSts_SysServSnsrForFCmptmtTType_read)
  CCMDcMotor_Runnable_sys/1(DcMotorCurPosVol_DcMotorCurPosVol_write) -> DcMotorCurPosVol_DcMotorCurPosVol/1
  CCMDcMotor_Runnable_sys/2(NvMBlockDcMtrCalDataArray_NvMBlockDcMtrCalDataArray_write) -> NvMBlockDcMtrCalDataArray_NvMBlockDcMtrCalDataArray/1
  CCMDcMotor_Runnable_sys/3(DcMotorCaliTyp_DcMotorCaliTyp_write) -> DcMotorCaliTyp_DcMotorCaliTyp/1
  BswLifeCycle_LifeCycle/1 -> CCMDcMotor_Runnable_sys/115(BswLifeCycle_LifeCycle_read)
  SbcWakeupSta_SbcWakeupSta/1 -> [SbcWakeupSta_SbcWakeupSta_Tag]
  [SbcWakeupSta_SbcWakeupSta_Tag] -> CCMDcMotor_Init/1(SbcWakeupSta_SbcWakeupSta_read)
  [SbcWakeupSta_SbcWakeupSta_Tag] -> CCMDcMotor_Runnable_sys/103(SbcWakeupSta_SbcWakeupSta_read)
  CCMDcMotor_DiagRunnable/1 -> CCMDcMotor_DiagRunnable_sys/Trigger
  CCMDcMotor_Runnable/1 -> CCMDcMotor_Runnable_sys/Trigger

== system CCMDcMotor/CCMDcMotor_DiagRunnable_sys ==
  function : TriggerPort  TriggerType=function-call  VariantControl=(inherit)  SampleTimeType=periodic  SampleTime=-1
  PA_NvMBlockDcMtrCalDataInit : Constant  Value=NvMBlockDcMtrCalDataInit
  Terminator_1 : Terminator
  wires:
  PA_NvMBlockDcMtrCalDataInit/1 -> Terminator_1/1

== system CCMDcMotor/CCMDcMotor_Init ==
  SbcWakeupSta_SbcWakeupSta_read : Inport
  Event Listener : EventListener  EventName=reset
  PA_NvMBlockDcMtrCalDataInit : Constant  Value=NvMBlockDcMtrCalDataInit
  Terminator_1 : Terminator
  Terminator_2 : Terminator
  wires:
  SbcWakeupSta_SbcWakeupSta_read/1 -> Terminator_1/1
  PA_NvMBlockDcMtrCalDataInit/1 -> Terminator_2/1
